simulink model "s_c_nbe_m1k_fulldb"  (R2016b) (continued)
== system s_c_nbe_m1k_fulldb == (continued)
  pipeline30/1(q) -> [force_gbe_txen]
  control/18(in_snap_stop_mux_sel) -> pipeline127/1(d)
  control/19(in_snap_reord_mux_we_sel) -> pipeline128/1(d)
  pipeline127/1(q) -> [snap_stop_mux_sel]
  pipeline128/1(q) -> [snap_reord_mux_we_sel]
  control/20(in_local_time_source) -> pipeline6/1(d)
  edge_detect6/1(out) -> pipeline164/1(d)
  pipeline164/1(q) -> [local_time_capture]
  pipeline6/1(q) -> [local_time_src]
  control/21(in_local_time_capture) -> edge_detect6/1(in)
  control/22(in_time_diff_check_en) -> pipeline62/1(d)
  pipeline62/1(q) -> [time_diff_check_en]
  control/23(in_auto_rst_enable) -> pipeline132/1(d)
  pipeline132/1(q) -> [auto_rst_enable]
  pipeline18/1(q) -> [pipeline_stall]
  control/24(in_pipeline_stall) -> pipeline18/1(d)
  Counter12/1 -> Slice13/1
  Slice13/1 -> edge_detect8/1(in)
  Logical15/1 -> Logical11/2, Counter12/1
  Logical11/1 -> [fault_detected]
  Register6/1 -> Inverter6/1
  Register/1 -> [synced], posedge_delay/1(In1)
  posedge_delay/1(Out1) -> [synced_delay]
  [board_in_fault] -> Inverter1/1
  Inverter1/1 -> Logical6/1
  [synced] -> Inverter2/1
  Inverter2/1 -> Counter1/2
  [pack_sync] -> Counter1/1
  Counter1/1 -> Slice16/1
  Slice16/1 -> edge_detect5/1(in)
  edge_detect8/1(out) -> Logical11/1
  [sys_relock] -> Delay57/1
  Inverter6/1 -> [board_in_fault]
  Delay57/1 -> Register6/2
  [synced_delay] -> edge_detect9/1(in)
  edge_detect9/1(out) -> Register6/3, Register6/1
  edge_detect5/1(out) -> [sync_timeout]
  [auto_rst_enable] -> Logical7/1
  [synced_delay] -> Logical7/2
  [fault_detected] -> Logical7/3
  [sync_timeout] -> Logical14/2
  [auto_rst_enable] -> Logical14/1
  Logical14/1 -> Logical8/2
  Logical7/1 -> Logical8/3
  pipeline23/1(q) -> [outfreq_stopmux]
  ct_debug/6(in_outfreq_stopmux) -> pipeline23/1(d)
  pipeline24/1(q) -> [out_stopmux]
  ct_debug/5(in_out_stopmux) -> pipeline24/1(d)
  pipeline55/1(q) -> [wrrd_stopmux]
  ct_debug/3(in_wrrd_stopmux) -> pipeline55/1(d)
  pipeline56/1(q) -> [in_stopmux]
  ct_debug/1(in_in_stopmux) -> pipeline56/1(d)
  pipeline122/1(q) -> [ct_snap_arm]
  pipeline22/1(q) -> edge_detect7/1
  ct_debug/8(in_snap_trig) -> pipeline22/1(d)
  ct_debug/7(in_snap_arm) -> pipeline122/1(d)
  edge_detect7/1 -> [ct_snap_trig]
  ct_debug/2(in_in_trigmux) -> pipeline150/1(d)
  pipeline150/1(q) -> [in_trigmux]
  ct_debug/4(in_obuf_stopmux) -> pipeline167/1(d)
  pipeline167/1(q) -> [obuf_stopmux]
  ct_debug/9(in_snap_wesel) -> pipeline81/1(d)
  pipeline81/1(q) -> [ct_snap_wesel]
  pipeline157/1(q) -> [ct_snap_trigsel]
  ct_debug/10(in_snap_trigsel) -> pipeline157/1(d)
  pipeline166/1(q) -> [ct_snap_circsel]
  ct_debug/11(in_snap_circsel) -> pipeline166/1(d)
  bus_create2/1(bus_out) -> [tvgtone_level0]
  bus_create3/1(bus_out) -> [tvgtone_level1]
  tvg_tone_levels/2(in_p0i) -> bus_create2/2(in2)
  tvg_tone_levels/3(in_p1r) -> bus_create3/1(in1)
  tvg_tone_levels/4(in_p1i) -> bus_create3/2(in2)
  tvg_tone_levels/1(in_p0r) -> bus_create2/1(in1)
  tvg_tone_targets/1(in_p0) -> Slice18/1
  tvg_tone_targets/2(in_p1) -> Slice19/1
  Constant51/1 -> tvg_tone_targets/1(sim_1)
  Constant52/1 -> tvg_tone_targets/2(sim_2)
  Constant37/1 -> tvg_tone_levels/4(sim_4)
  Constant8/1 -> tvg_tone_levels/1(sim_1)
  Constant27/1 -> tvg_tone_levels/3(sim_3)
  Constant9/1 -> tvg_tone_levels/2(sim_2)
  we_delay49/1(q) -> [ct_tag_insert_sel]
  edge_detect2/1 -> [ct_rst_err_latch]
  we_delay47/1(q) -> edge_detect2/1
  we_delay48/1(q) -> [ct_fake_data_error]
  we_delay13/1(q) -> [ct_tvg_en]
  we_delay50/1(q) -> [ct_tvg_en2]
  Constant12/1 -> ct_control0/5(sim_5)
  Constant21/1 -> ct_control0/4(sim_4)
  Constant20/1 -> ct_control0/3(sim_3)
  Constant22/1 -> ct_control0/2(sim_2)
  Constant15/1 -> ct_control0/1(sim_1)
  ct_control0/1(in_tvg_en) -> we_delay13/1(d)
  ct_control0/2(in_tvg_en2) -> we_delay50/1(d)
  ct_control0/3(in_rst_err_latch) -> we_delay47/1(d)
  ct_control0/4(in_fake_error) -> we_delay48/1(d)
  ct_control0/5(in_tag_insert) -> we_delay49/1(d)
  we_delay126/1(q) -> [obuf_read_gap]
  ct_control0/6(in_obuf_read_gap) -> we_delay126/1(d)
  Constant30/1 -> ct_control0/6(sim_6)
  we_delay41/1(q) -> [ct_num_xeng]
  we_delay112/1(q) -> [ct_num_xeng_recip]  "NUM_X_RECIP"
  ct_control1/2(in_num_x_recip) -> we_delay112/1(d)
  ct_control1/1(in_num_x) -> we_delay41/1(d)
  we_delay114/1(q) -> [ct_x_per_board]
  ct_control1/3(in_x_per_board) -> we_delay114/1(d)
  we_delay113/1(q) -> [ct_chans_per_board]
  ct_control2/1(in_chans_per_x) -> we_delay39/1(d)
  ct_control2/2(in_chans_per_board) -> we_delay113/1(d)
  we_delay115/1(q) -> [ct_x_per_board_recip]
  ct_control1/4(in_x_per_board_recip) -> we_delay115/1(d)
  we_delay38/1(q) -> [ct_num_x_boards]
  ct_control3/1(in_num_x_boards) -> we_delay38/1(d)
  we_delay37/1(q) -> [ct_num_x_boards_recip]
  ct_control3/2(in_num_x_boards_recip) -> we_delay37/1(d)
  ct_control3/3(in_chans_per_x_recip) -> we_delay124/1(d)
  Constant49/1 -> ct_control1/1(sim_1)
  Constant53/1 -> ct_control1/2(sim_2)
  Constant33/1 -> ct_control1/3(sim_3)
  Constant23/1 -> ct_control2/1(sim_1)
  Constant50/1 -> ct_control2/2(sim_2)
  Constant54/1 -> ct_control1/4(sim_4)
  Constant32/1 -> ct_control3/1(sim_1)
  Constant14/1 -> ct_control3/2(sim_2)
  Constant44/1 -> ct_control3/3(sim_3)
  Slice18/1 -> [tvgtone_p0]
  Slice19/1 -> [tvgtone_p1]
  Slice36/1 -> we_delay116/1(d)
  Constant38/1 -> ct_control4/1(sim_1)
  ct_control4/1(in_ct_board_offset) -> Slice36/1
  ct_control5/1(in_ct_freq_gen_offset) -> we_delay117/1(d)
  Constant39/1 -> ct_control5/1(sim_1)
  we_delay116/1(q) -> [ct_board_hmc_offset]
  we_delay117/1(q) -> [ct_freq_gen_offset]
  we_delay124/1(q) -> [ct_chans_per_x_recip]
  we_delay39/1(q) -> [ct_chans_per_x]  "PER_X"
  pipeline196/1(q) -> ddc_dvalid_cnt/1(out_reg)
  pipeline197/1(q) -> ddc_sync_cnt/1(out_reg)
  pipeline198/1(q) -> DDC/8(scale1)
  pipeline199/1(q) -> DDC/5(scale0)
  trig_time_msw/1(in_msw) -> pipeline213/1(d)
  trig_time_lsw/1(in_lsw) -> pipeline214/1(d)
  Concat13/1 -> Relational10/1
  pipeline213/1(q) -> Concat13/1
  pipeline214/1(q) -> Concat13/2
  [time80_48] -> Relational10/2
  pipeline215/1(q) -> bus_expand18/1(bus_in)
  [valid80] -> Slice5/1
  Slice14/1 -> pipeline211/1(d)
  [time80_48] -> Slice14/1
  pipeline211/1(q) -> snap_adc0/13(extra_timestamp)
  [d80_adc0] -> pipeline215/1(d)
  bus_expand18/4(out5) -> snap_adc0/4(in_p0_d3)
  bus_expand18/5(out4) -> snap_adc0/5(in_p0_d4)
  bus_expand18/1(msb_out8) -> Gateway Out3/1, snap_adc0/1(in_p0_d0)
  bus_expand18/2(out7) -> Gateway Out23/1, snap_adc0/2(in_p0_d1)
  bus_expand18/3(out6) -> snap_adc0/3(in_p0_d2)
  bus_expand18/6(out3) -> snap_adc0/6(in_p0_d5)
  bus_expand18/7(out2) -> snap_adc0/7(in_p0_d6)
  bus_expand18/8(lsb_out1) -> snap_adc0/8(in_p0_d7)
  pipeline202/1(q) -> snap_adc0/11(we)
  Relational10/1 -> Logical12/1
  [valid80] -> pipeline162/1(d)
  Logical12/1 -> snap_adc0/12(trig)
  pipeline162/1(q) -> Logical12/2
  [pfbin_sync] -> Delay21/1
  [ddc_sync_in] -> pipeline97/1(d)
  pipeline68/1(q) -> pipeline66/1(d)
  pipeline83/1(q) -> pipeline59/1(d)
  Slice5/1 -> pipeline68/1(d), pipeline202/1(d)
  [sync80] -> pipeline83/1(d)
  [ddc_pol1_in] -> pipeline93/1(d)
  Slice17/1 -> pipeline121/1(d)
  Slice15/1 -> Convert1/1
  [local_time_capture] -> Delay31/1
  pipeline19/1(q) -> Slice17/1, Slice15/1
  [local_time_src] -> Mux/1
  [time80_48] -> Delay14/1
  [valid80] -> Delay14/2
  Delay14/1 -> Register1/1, AddSub/2
  [pfb_time] -> Delay10/1
  [pfb_dv] -> Delay10/2
  Delay11/1 -> Register3/1, AddSub/1
  [flag_dvalid] -> Delay11/2
  [pack_sync_time] -> Delay11/1
  Convert1/1 -> pipeline123/1(d)
  AddSub/1 -> pipeline140/1(d), Relational4/1
  Relational4/1 -> Logical/1
  Logical/1 -> [time_diff_error]
  time_check/1(in_max_difference) -> pipeline139/1(d)
  [time_diff_check_en] -> Logical/2
  Delay31/1 -> Register3/2, Register2/2, Register4/2, Register1/2
  Delay10/1 -> Register2/1
  [spead_timestamp] -> Register4/1
  Register4/1 -> Mux/2
  Register1/1 -> Mux/3
  Register2/1 -> Mux/4
  Register3/1 -> Mux/5
  Mux/1 -> pipeline19/1(d)
  [cnt_rst] -> Subsystem2/1(rst)
  [synced] -> Delay38/1
  [sync_armed] -> Delay39/1
  [board_in_fault] -> Delay48/1
  [q_sync] -> Subsystem19/5(in3)
  [pfb_sync] -> Subsystem19/3(in1)
  [fdfs_sync] -> Subsystem19/4(in2)
  [sync80] -> Subsystem19/2(in0)
  [cnt_rst] -> Subsystem19/1(rst)
  Subsystem19/2(out1) -> pipeline35/1(d)
  Subsystem19/1(out0) -> pipeline34/1(d)
  Subsystem19/3(out2) -> pipeline36/1(d)
  [pack_sync] -> Subsystem12/4(in2)
  [flag_sync] -> Subsystem12/3(in1)
  [ct_sync] -> Subsystem12/2(in0)
  [cnt_rst] -> Subsystem12/1(rst)
  [synced_delay] -> Subsystem2/2(armed)
  [sync_timeout] -> Subsystem2/3(in1)
  [time_diff_error] -> Subsystem2/4(in2)
  [up_timestep_error] -> Subsystem2/5(in3)
  [cd_error] -> Subsystem2/6(in4)
  [ct_error] -> Subsystem2/7(in5)
  Delay48/1 -> pipeline5/1(d)
  Delay38/1 -> pipeline10/1(d)
  Delay39/1 -> pipeline11/1(d)
  Subsystem12/1(out0) -> pipeline16/1(d)
  Subsystem12/2(out1) -> pipeline20/1(d)
  Subsystem12/3(out2) -> pipeline21/1(d)
  Subsystem2/1(Out1) -> pipeline25/1(d)
  Subsystem2/2(Out2) -> pipeline26/1(d)
  Subsystem2/3(Out3) -> pipeline27/1(d)
  [synced_delay] -> Delay58/1
  Delay58/1 -> pipeline4/1(d)
  Subsystem19/4(out3) -> pipeline37/1(d)
  Subsystem2/4(Out4) -> pipeline32/1(d)
  Subsystem2/5(Out5) -> pipeline33/1(d)
  [clr_status] -> Subsystem3/1(rst)
  Slice6/1 -> Subsystem3/2(in0)
  [pfb_of] -> Slice6/1, Slice20/1
  Slice20/1 -> Subsystem3/3(in1)
  [pfb_sync] -> Subsystem3/4(in2)
  Subsystem3/3(out2) -> pipeline193/1(d)
  Subsystem3/1(out0) -> pipeline195/1(d)
  Subsystem3/2(out1) -> pipeline194/1(d)
  Constant17/1 -> [pfb_of]
  Counter7/1 -> pipeline191/1(d)
  [pfb_sync] -> pipeline126/1(d)
  [cnt_rst] -> pipeline129/1(d)
  pipeline191/1(q) -> nb_sync_cnt/1(out_reg)
  pipeline193/1(q) -> pfb_status/3(out_sync_cnt)
  pipeline194/1(q) -> pfb_status/2(out_pol1_or_err_cnt)
  pipeline195/1(q) -> pfb_status/1(out_pol0_or_err_cnt)
  Delay72/1 -> [flag_sync]
  Delay75/1 -> [flag_misc]
  Delay73/1 -> [flag_dvalid]
  Delay74/1 -> [flag_time]
  Delay43/1 -> time_gen1/4(rd_sync_in)
  Delay59/1 -> time_gen1/6(rd_misc_in)
  Delay/1 -> Delay43/1  "gapsync"
  Delay3/1 -> Delay47/1  "gapdata"
  Mux1/1 -> Delay59/1  "gapvalid"
  [time80] -> Slice2/1
  Delay47/1 -> time_gen1/5(rd_en)
  time_gen1/1(rd_sync_out) -> Delay72/1
  time_gen1/4(rd_misc_out) -> Delay75/1
  time_gen1/2(rd_dvalid) -> Delay73/1
  time_gen1/3(rd_val_out) -> Delay74/1
  Slice2/1 -> time_gen1/3(wr_val_in)
  [ct_tvg_en2] -> Mux1/1
  Delay17/1 -> Mux1/2
  Delay18/1 -> tvg/1(sync), Delay/1
  Delay16/1 -> tvg/2(dv), Delay3/1
  tvg/1(data256) -> Mux1/3
  [flag_dvalid] -> Subsystem13/1(In1)
  Subsystem13/1(Out1) -> pipeline186/1(d)
  [s256_v] -> Subsystem11/1(In1)
  Subsystem11/1(Out1) -> pipeline187/1(d)
  pipeline38/1(q) -> Convert3/1
  pipeline46/1(q) -> [ip_per_x]
  pipeline47/1(q) -> [num_x]
  x_setup/1(in_f_per_x) -> pipeline38/1(d)
  x_setup/2(in_ip_per_x) -> pipeline46/1(d)
  x_setup/3(in_num_x) -> pipeline47/1(d)
  pipeline52/1(q) -> [pack_snap_arm]
  x_setup/4(in_snap_arm) -> pipeline52/1(d)
  pipeline48/1(q) -> [pktstart_sel]
  x_setup/5(in_pktstart_mux) -> pipeline48/1(d)
  [s256_v] -> pack_block_check/1(dv)
  [cnt_rst] -> Subsystem14/1(rst)
  Subsystem14/1(out0) -> pipeline188/1(d)
  pack_block_check/1(error) -> [pack_dv_err], Subsystem14/2(in0)
  pipeline49/1(q) -> [pack_mux]
  x_setup/6(in_pack_mux) -> pipeline49/1(d)
  Convert3/1 -> [f_per_x]
  [ct_d256] -> Delay17/1
  [ct_dv] -> Delay16/1
  [ct_sync] -> Delay18/1
  x_setup/7(in_pktoffset_sel) -> pipeline54/1(d)
  pipeline54/1(q) -> [pktoffset_sel]
  iptx_base/1(in_reg) -> pipeline53/1(d)
  pipeline53/1(q) -> [tx_ip_base]
  tx_metadata/1(in_porttx) -> pipeline50/1(d)
  tx_metadata/2(in_board_id) -> pipeline51/1(d)
  pipeline50/1(q) -> [tx_port]
  pipeline51/1(q) -> [tx_board_id]
  Delay64/1 -> Delay68/1
  Delay4/1 -> Delay5/1
  Delay5/1 -> Delay60/1
  Delay60/1 -> Delay64/1
  Delay68/1 -> [flag_pktstart]
  Delay1/1 -> Delay6/1
  Delay6/1 -> Delay61/1
  Delay65/1 -> Delay69/1
  Delay69/1 -> [flag_pktfreq]
  Delay61/1 -> Delay65/1
  Delay15/1 -> Delay7/1
  Delay7/1 -> Delay62/1
  Delay62/1 -> Delay66/1
  Delay66/1 -> Delay70/1
  Delay70/1 -> [flag_pktxindex]
  Delay19/1 -> Delay20/1
  Delay20/1 -> Delay63/1
  Delay63/1 -> Delay67/1
  Delay67/1 -> Delay71/1
  Delay71/1 -> [flag_pktxoffset]
  [ct_pkt_x_index] -> Delay15/1
  [ct_pkt_x_offset] -> Delay19/1
  [ct_pktfreq] -> Delay1/1
  [ct_pktstart] -> Delay4/1
  [flag_sync] -> packetiser/1(sync_in)
  [flag_dvalid] -> packetiser/2(en)
  [flag_pktstart] -> packetiser/5(pkt_start)
  [flag_pktxoffset] -> packetiser/7(pkt_x_offset)
  [tx_ip_base] -> packetiser/9(base_ip)
  [tx_port] -> packetiser/10(base_port)
  Constant3/1 -> concat1/2
  [flag_time] -> concat1/1
  [flag_misc] -> packetiser/3(din)
  concat1/1 -> [pack_sync_time], packetiser/4(timestamp)
  Delay82/1 -> [s256_d]
  Delay83/1 -> [s256_v]
  Delay84/1 -> [s256_eof]
  Delay85/1 -> [s256_of]
  Delay86/1 -> [s256_ip]
  Delay87/1 -> [s256_port]
  Delay88/1 -> [pack_sync]
  packetiser/1(dout) -> Delay82/1
  packetiser/2(dvalid) -> Delay83/1
  packetiser/3(eof) -> Delay84/1
  packetiser/4(overflow) -> Delay85/1
  packetiser/5(destination_ip) -> Delay86/1
  packetiser/6(destination_port) -> Delay87/1
  packetiser/7(sync) -> Delay88/1
  [flag_pktfreq] -> Delay77/1
  Delay89/1 -> [s256_pktfreq]
  Delay77/1 -> Delay89/1
  [flag_pktxindex] -> Delay78/1
  Delay78/1 -> Delay90/1
  Delay90/1 -> [s256_pktxindex]
  [flag_pktxoffset] -> Delay79/1
  Delay79/1 -> Delay91/1
  Delay91/1 -> [s256_pktxoffset]
  [tx_board_id] -> packetiser/8(board_id)
  [flag_pktstart] -> Delay80/1
  Delay80/1 -> Delay92/1
  Delay92/1 -> [s256_pktstart]
  [sync80] -> time_gen1/1(wr_sync_in)
  [valid80] -> time_gen1/2(wr_en)
  [time80_48] -> Subsystem/1(time)
  [sync80] -> Subsystem/2(sync)
  [valid80] -> Subsystem/3(dv)
  Subsystem/1(msw) -> pipeline184/1(d)
  Subsystem/2(ls32) -> pipeline185/1(d)
  [flag_pktxindex] -> packetiser/6(pkt_x_index)
  [flag_time] -> Delay81/1
  Delay81/1 -> Delay93/1
  Delay93/1 -> [s256_time]
  time_gen1/5(epoch) -> Delay76/1
  Slice4/1 -> pipeline182/1(d)
  Slice11/1 -> pipeline183/1(d)
  Delay76/1 -> Concat10/1
  Constant16/1 -> Concat10/2
  Concat10/1 -> Slice4/1, Slice11/1, [flag_epoch]
  [flag_pktfreq] -> Subsystem27/3(value)
  [cnt_rst] -> Subsystem27/1(rst)
  [flag_dvalid] -> Subsystem27/2(en)
  Subsystem27/1(current) -> pipeline170/1(d)
  Subsystem27/3(min) -> pipeline172/1(d)
  Subsystem27/2(max) -> pipeline171/1(d)
  [flag_pktxindex] -> Subsystem28/3(value)
  [cnt_rst] -> Subsystem28/1(rst)
  [flag_dvalid] -> Subsystem28/2(en)
  Subsystem28/1(current) -> pipeline173/1(d)
  Subsystem28/3(min) -> pipeline175/1(d)
  Subsystem28/2(max) -> pipeline174/1(d)
  [flag_pktxoffset] -> Subsystem29/3(value)
  [cnt_rst] -> Subsystem29/1(rst)
  [flag_dvalid] -> Subsystem29/2(en)
  Subsystem29/1(current) -> pipeline176/1(d)
  Subsystem29/3(min) -> pipeline178/1(d)
  Subsystem29/2(max) -> pipeline177/1(d)
  [flag_time] -> Subsystem30/3(value)
  [cnt_rst] -> Subsystem30/1(rst)
  [flag_pktstart] -> Subsystem30/2(en)
  Subsystem30/1(current) -> pipeline179/1(d)
  Subsystem30/3(min) -> pipeline181/1(d)
  Subsystem30/2(max) -> pipeline180/1(d)
  pipeline170/1(q) -> flag_pktfreq_stats/1(out_current)
  pipeline171/1(q) -> flag_pktfreq_stats/2(out_max)
  pipeline172/1(q) -> flag_pktfreq_stats/3(out_min)
  pipeline175/1(q) -> flag_pktxindex_stats/3(out_min)
  pipeline174/1(q) -> flag_pktxindex_stats/2(out_max)
  pipeline173/1(q) -> flag_pktxindex_stats/1(out_current)
  pipeline176/1(q) -> flag_pktxoffset_stats/1(out_current)
  pipeline177/1(q) -> flag_pktxoffset_stats/2(out_max)
  pipeline178/1(q) -> flag_pktxoffset_stats/3(out_min)
  pipeline181/1(q) -> flag_time_stats/3(out_min)
  pipeline180/1(q) -> flag_time_stats/2(out_max)
  pipeline179/1(q) -> flag_time_stats/1(out_current)
  pipeline182/1(q) -> flag_epoch_lsw/1(out_reg)
  pipeline183/1(q) -> flag_epoch_msw/1(out_reg)
  pipeline184/1(q) -> sync_time_msw/1(out_reg)
  pipeline185/1(q) -> sync_time_lsw/1(out_reg)
  pipeline186/1(q) -> pack_in_dv_rate/1(out_dv_rate)
  pipeline187/1(q) -> pack_out_dv_rate/1(out_dv_rate)
  pipeline188/1(q) -> pack_dv_err/1(out_dvblock_err_cnt)
  [pfbin_sync] -> Logical9/2
  [sys_rst] -> Logical9/1
  Logical9/1 -> pipeline147/1(d)
  [osc_cos_ddc0] -> pipeline92/1(d)
  [osc_cos_ddc0] -> pipeline94/1(d)
  [pfbin_d0] -> Delay9/1
  [pfbin_dv] -> Delay2/1
  Delay21/1 -> pipeline144/1(d)
  [ddc_pol0] -> nb_pfb/2(pol0)
  [ddc_pol1] -> nb_pfb/3(pol1)
  nb_pfb/2(pol0_out) -> [pfb_d144_0]
  nb_pfb/3(pol1_out) -> [pfb_d144_1]
  [ddc_sync] -> nb_pfb/1(sync)
  nb_pfb/1(sync_out) -> [pfb_sync]
  [ddc_dvalid] -> nb_pfb/4(dvalid)
  nb_pfb/4(dv_out) -> [pfb_dv]
  [ddc_dv_in] -> pipeline96/1(d)
  [ddc_pol0_in] -> pipeline95/1(d)
  [pfbin_d1] -> Delay22/1
  [pfbin_dv] -> cwg1/6(en)
  Delay22/1 -> pipeline149/1(d)
  Gateway Out3/1 -> To Workspace4/1
  Gateway Out23/1 -> To Workspace23/1
  tl_fd0/2(enable) -> phase_compensation0/5(load_value)
  tl_fd1/1(value) -> phase_compensation1/4(delay_value)
  tl_fd1/2(enable) -> phase_compensation1/5(load_value)
  tl_fd0/1(value) -> phase_compensation0/4(delay_value)
  [clr_status] -> tl_fd1/1(rst), tl_fd0/1(rst)
  phase_compensation0/1(sync_out) -> [fdfs_sync]
  phase_compensation1/1(sync_out) -> Terminator24/1
  phase_compensation0/2(dout) -> [fdfs_d144_0]
  phase_compensation1/2(dout) -> [fdfs_d144_1]
  phase_compensation0/3(dvalid) -> [fdfs_dv]
  phase_compensation1/3(dvalid) -> Terminator25/1
  [ld_val_fd0] -> tl_fd0/3(load_value)
  [ld_val_fd1] -> tl_fd1/3(load_value)
  bus_expand16/1(msb_out2) -> tl_fd0/5(din)
  tl_fd0/5(dvalid) -> phase_compensation0/3(en)
  tl_fd0/3(sync_out) -> phase_compensation0/1(sync_in)
  tl_fd0/4(dout) -> phase_compensation0/2(din)
  tl_fd1/4(dout) -> phase_compensation1/2(din)
  bus_expand16/2(lsb_out1) -> tl_fd1/5(din)
  Constant66/1 -> Concat2/2
  Concat2/1 -> tl_fd1/2(current_time), tl_fd0/2(current_time), [pfb_time]
  Counter2/1 -> pipeline41/1(d)
  Constant65/1 -> eq1/2(data_in)
  Constant24/1 -> eq1/3(we)
  Delay53/1 -> quant1/1(sync_in)
  Delay37/1 -> quant1/2(din)
  eq1/1(data_out) -> we_delay28/1(d)
  Delay50/1 -> quant1/3(en)
  Counter4/1 -> pipeline29/1(d)
  Constant63/1 -> eq0/2(data_in)
  Constant64/1 -> eq0/3(we)
  Delay51/1 -> quant0/1(sync_in)
  Delay35/1 -> quant0/2(din)
  Delay52/1 -> quant0/3(en)
  quant0/1(sync_out) -> [q_sync]
  quant0/2(dout) -> [q_d64_0]
  quant0/3(dvalid) -> [q_dv]
  quant0/4(of) -> [q_of0]
  quant1/2(dout) -> [q_d64_1]
  quant1/1(sync_out) -> Terminator19/1
  quant1/3(dvalid) -> Terminator21/1
  quant1/4(of) -> [q_of1]
  pipeline29/1(q) -> eq0/1(addr)
  pipeline41/1(q) -> eq1/1(addr)
  [fdfs_sync] -> Delay51/1, pipeline45/1(d)
  [fdfs_d144_0] -> Delay35/1
  [fdfs_dv] -> Delay52/1, pipeline42/1(d)
  pipeline42/1(q) -> Counter4/2
  pipeline45/1(q) -> Counter4/1
  pipeline189/1(q) -> Counter2/2
  [fdfs_sync] -> Delay53/1, pipeline200/1(d)
  [fdfs_d144_1] -> Delay37/1
  [fdfs_dv] -> Delay50/1, pipeline189/1(d)
  pipeline200/1(q) -> Counter2/1
  [clr_status] -> Subsystem15/1(rst)
  tl_fd0/7(misco) -> Terminator3/1
  tl_fd0/6(time) -> Terminator4/1
  tl_fd1/6(time) -> Terminator17/1
  tl_fd1/3(sync_out) -> phase_compensation1/1(sync_in)
  tl_fd1/5(dvalid) -> phase_compensation1/3(en)
  tl_fd1/7(misco) -> Terminator20/1
  Constant26/1 -> tl_fd0/7(misci)
  Constant29/1 -> tl_fd1/7(misci)
  time_gen/1(rd_sync_out) -> tl_fd1/4(sync_in), tl_fd0/4(sync_in)
  time_gen/2(rd_dvalid) -> tl_fd1/6(en), tl_fd0/6(en)
  time_gen/3(rd_val_out) -> Concat2/1
  time_gen/4(rd_misc_out) -> bus_expand16/1(bus_in)
  [sync80] -> pipeline207/1(d)
  [valid80] -> pipeline208/1(d)
  [pfb_sync] -> pipeline210/1(d)
  [pfb_dv] -> pipeline212/1(d)
  [pfb_d144_1] -> pipeline231/1(d)
  [pfb_d144_0] -> pipeline228/1(d)
  Concat1/1 -> time_gen/6(rd_misc_in)
  [time80] -> pipeline209/1(d)
  Subsystem15/1(out0) -> we_delay18/1(d)
  Relational/1 -> Subsystem15/2(in0)
  Constant4/1 -> Relational/2
  [q_of0] -> Relational/1
  Relational3/1 -> Subsystem15/3(in1)
  [q_of1] -> Relational3/1
  Constant25/1 -> Relational3/2
  Subsystem15/2(out1) -> we_delay19/1(d)
  [tl_cd0_arm] -> tl_fd0/8(arm)
  [tl_cd0_load_immediate] -> tl_fd0/9(load_immediate)
  [tl_cd0_load_time] -> tl_fd0/10(load_time)
  [tl_cd1_arm] -> tl_fd1/8(arm)
  [tl_cd1_load_immediate] -> tl_fd1/9(load_immediate)
  [tl_cd1_load_time] -> tl_fd1/10(load_time)
  pipeline207/1(q) -> time_gen/1(wr_sync_in)
  pipeline208/1(q) -> time_gen/2(wr_en)
  pipeline209/1(q) -> time_gen/3(wr_val_in)
  pipeline210/1(q) -> time_gen/4(rd_sync_in)
  pipeline212/1(q) -> time_gen/5(rd_en)
  pipeline228/1(q) -> Concat1/1
  pipeline231/1(q) -> Concat1/2
  Concat4/1 -> pipeline133/1(d)
  Concat9/1 -> pipeline130/1(d)
  fd0_phase/1(in_initial) -> Reinterpret10/1
  fd0_delta_delay/1(in_delta) -> Reinterpret9/1
  fd0_phase/2(in_delta) -> Reinterpret11/1
  Reinterpret16/1 -> Concat4/1
  Reinterpret9/1 -> Concat4/2
  Reinterpret10/1 -> Concat4/3
  Reinterpret11/1 -> Concat4/4
  fd1_phase/1(in_initial) -> Reinterpret13/1
  fd1_delta_delay/1(in_delta) -> Reinterpret12/1
  fd1_phase/2(in_delta) -> Reinterpret14/1
  Reinterpret12/1 -> Concat9/2
  Reinterpret13/1 -> Concat9/3
  Reinterpret14/1 -> Concat9/4
  Reinterpret15/1 -> Concat9/1
  fd0_delay/1(in_initial) -> Reinterpret16/1
  fd1_delay/1(in_initial) -> Reinterpret15/1
  [ct_dv] -> Subsystem21/1(In1)
  Subsystem21/1(Out1) -> we_delay22/1(d)
  [ct_pktstart] -> we_delay3/1(d)
  [ct_dv] -> we_delay4/1(d)
  we_delay3/1(q) -> pktlen_check1/1(pkt_start)
  we_delay4/1(q) -> dv_block_check1/1(dv), pktlen_check1/2(dv)
  pktlen_check1/1(error) -> we_delay6/1(d)
  dv_block_check1/1(error) -> we_delay5/1(d)
  [cnt_rst] -> Subsystem20/1(rst)
  we_delay6/1(q) -> [ct_pktlen_err], Subsystem20/2(in0)
  we_delay5/1(q) -> [ct_dvblk_err], Subsystem20/3(in1)
  Subsystem20/1(out0) -> we_delay20/1(d)
  Subsystem20/2(out1) -> we_delay21/1(d)
  [q_dv] -> Subsystem18/1(In1)
  Subsystem18/1(Out1) -> we_delay23/1(d)
  [q_sync] -> we_delay14/1(d)
  [q_dv] -> we_delay15/1(d)
  [q_d64_0] -> we_delay16/1(d)
  [q_d64_1] -> we_delay17/1(d)
  hmc_ct/1(out_sync) -> we_delay12/1(d)
  hmc_ct/2(out_dv) -> we_delay11/1(d)
  hmc_ct/3(out_d256) -> we_delay10/1(d)
  hmc_ct/4(pkt_start) -> we_delay9/1(d)
  hmc_ct/5(freq_id) -> we_delay8/1(d)
  hmc_ct/6(x_index) -> we_delay7/1(d)
  hmc_ct/7(x_pkt_index) -> we_delay2/1(d)
  hmc_ct/8(error0) -> we_delay1/1(d)
  [cnt_rst] -> Subsystem16/1(rst)
  [q_sync] -> Subsystem16/2(in0)
  Constant67/1 -> Subsystem16/3(in1)
  Constant68/1 -> Subsystem16/4(in2)
  [ct_sync] -> Subsystem16/5(in3)
  Subsystem16/1(out0) -> we_delay24/1(d)
  Subsystem16/2(out1) -> we_delay25/1(d)
  Subsystem16/3(out2) -> we_delay26/1(d)
  Subsystem16/4(out3) -> we_delay27/1(d)
  Convert2/1 -> [cd_bypass]
  pipeline59/1(q) -> snap_adc0/9(in_sync)
  pipeline66/1(q) -> snap_adc0/10(in_dv)
  pipeline8/1(q) -> [unpack_dv]
  pipeline17/1(q) -> [unpack_timestamp]
  pipeline14/1(q) -> [tvg_adc0_en]
  pipeline72/1(q) -> bus_expand4/1(bus_in)
  From8/1 -> Slice3/1
  Slice1/1 -> pipeline71/1(d)
  From33/1 -> Slice1/1
  pipeline71/1(q) -> snap_adc1/13(extra_timestamp)
  From35/1 -> pipeline72/1(d)
  bus_expand4/4(out5) -> snap_adc1/4(in_p0_d3)
  bus_expand4/5(out4) -> snap_adc1/5(in_p0_d4)
  bus_expand4/1(msb_out8) -> snap_adc1/1(in_p0_d0)
  bus_expand4/2(out7) -> snap_adc1/2(in_p0_d1)
  bus_expand4/3(out6) -> snap_adc1/3(in_p0_d2)
  bus_expand4/6(out3) -> snap_adc1/6(in_p0_d5)
  bus_expand4/7(out2) -> snap_adc1/7(in_p0_d6)
  bus_expand4/8(lsb_out1) -> snap_adc1/8(in_p0_d7)
  pipeline67/1(q) -> snap_adc1/11(we)
  pipeline77/1(q) -> pipeline76/1(d)
  pipeline79/1(q) -> snap_adc1/12(trig), pipeline75/1(d)
  Slice3/1 -> pipeline67/1(d), pipeline77/1(d)
  From34/1 -> pipeline79/1(d)
  pipeline75/1(q) -> snap_adc1/9(in_sync)
  pipeline76/1(q) -> snap_adc1/10(in_dv)
  pipeline13/1(q) -> [osc_cos_ddc0]
  Gateway Out5/1 -> To Workspace5/1
  [q_d64_0] -> bus_expand31/1(bus_in)
  [q_dv] -> Gateway Out5/1
  Gateway Out6/1 -> To Workspace6/1
  [q_sync] -> Gateway Out6/1
  Gateway Out7/1 -> To Workspace7/1
  bus_expand31/1(msb_out8) -> Gateway Out7/1
  Gateway Out8/1 -> To Workspace8/1
  Gateway Out9/1 -> To Workspace9/1
  bus_expand31/3(out6) -> Gateway Out9/1
  Gateway Out10/1 -> To Workspace10/1
  bus_expand31/5(out4) -> Gateway Out10/1
  Gateway Out11/1 -> To Workspace11/1
  bus_expand31/7(out2) -> Gateway Out11/1
  Gateway Out12/1 -> To Workspace12/1
  bus_expand31/4(out5) -> Gateway Out12/1
  Gateway Out13/1 -> To Workspace13/1
  bus_expand31/6(out3) -> Gateway Out13/1
  Gateway Out14/1 -> To Workspace14/1
  bus_expand31/8(lsb_out1) -> Gateway Out14/1
  bus_expand31/2(out7) -> Gateway Out8/1
  Gateway Out20/1 -> To Workspace20/1
  bus_expand32/1(msb_out8) -> Gateway Out20/1
  Gateway Out21/1 -> To Workspace15/1
  Gateway Out22/1 -> To Workspace16/1
  bus_expand32/3(out6) -> Gateway Out22/1
  Gateway Out15/1 -> To Workspace17/1
  bus_expand32/5(out4) -> Gateway Out15/1
  Gateway Out16/1 -> To Workspace18/1
  bus_expand32/7(out2) -> Gateway Out16/1
  Gateway Out17/1 -> To Workspace19/1
  bus_expand32/4(out5) -> Gateway Out17/1
  Gateway Out18/1 -> To Workspace21/1
  bus_expand32/6(out3) -> Gateway Out18/1
  Gateway Out19/1 -> To Workspace22/1
  bus_expand32/8(lsb_out1) -> Gateway Out19/1
  bus_expand32/2(out7) -> Gateway Out21/1
  [fdfs_d144_0] -> bus_expand32/1(bus_in)
  Gateway Out24/1 -> To Workspace24/1
  [fdfs_dv] -> Gateway Out24/1
  Gateway Out25/1 -> To Workspace25/1
  [fdfs_sync] -> Gateway Out25/1
  eq0/1(data_out) -> we_delay29/1(d)
  impulse_gen0/1(sync_out) -> [sync80]
  impulse_gen0/3(dvalid) -> [valid80]
  impulse_gen0/2(dout) -> [d80_adc0]
  [ld_val0] -> pipeline296/1(d)
  [clr_status] -> pipeline278/1(d), pipeline298/1(d)
  tl_cd0/6(time) -> [time80_tl_cd0]
  Constant74/1 -> Concat15/2
  Concat15/1 -> pipeline277/1(d), pipeline297/1(d)
  [time80] -> Concat15/1
  pipeline298/1(q) -> tl_cd0/1(rst)
  pipeline297/1(q) -> tl_cd0/2(current_time)
  pipeline296/1(q) -> tl_cd0/3(load_value)
  pipeline295/1(q) -> tl_cd0/4(sync_in)
  pipeline294/1(q) -> tl_cd0/5(din)
  pipeline299/1(q) -> tl_cd0/6(en)
  [sync80] -> pipeline283/1(d), pipeline295/1(d)
  [valid80] -> pipeline281/1(d), pipeline299/1(d)
  Constant13/1 -> tl_cd0/7(misci)
  tl_cd0/7(misco) -> Terminator6/1
  tl_cd0/3(sync_out) -> [sync_tl_cd0]
  tl_cd0/5(dvalid) -> [dvalid_tl_cd0]
  tl_cd0/4(dout) -> [dout_tl_cd0]
  tl_cd0/2(enable) -> [en_tl_cd0]
  tl_cd0/1(value) -> [delay_tl_cd0]
  pipeline307/1(q) -> tl_cd0/10(load_time)
  pipeline306/1(q) -> tl_cd0/9(load_immediate)
  pipeline305/1(q) -> tl_cd0/8(arm)
  [tl_cd0_arm] -> pipeline305/1(d)
  [tl_cd0_load_immediate] -> pipeline306/1(d)
  [tl_cd0_load_time] -> pipeline307/1(d)
  [d80_adc0] -> pipeline294/1(d)
  [dvalid_tl_cd0] -> Delay117/1
  [sync_tl_cd0] -> Delay120/1
  [en_tl_cd0] -> Delay115/1
  [delay_tl_cd0] -> Delay116/1
  Concat5/1 -> time_gen_cd/6(rd_misc_in)
  time_gen_cd/4(rd_misc_out) -> bus_expand7/1(bus_in)
  [sync_tl_cd0] -> time_gen_cd/1(wr_sync_in)
  [dvalid_tl_cd0] -> time_gen_cd/2(wr_en)
  [hmc_sync_p0] -> time_gen_cd/4(rd_sync_in)
  [hmc_dvalid] -> time_gen_cd/5(rd_en)
  time_gen_cd/1(rd_sync_out) -> [tg_rd_sync]
  time_gen_cd/2(rd_dvalid) -> [tg_rd_dvalid]
  [dvalid_tl_bs0] -> wb_prog_delay/4(en)
  [delay_tl_bs0] -> wb_prog_delay/2(delay_val)
  [delay_tl_sync0] -> wb_prog_delay/1(sync)
  [en_tl_bs0] -> wb_prog_delay/5(load_val)
  wb_prog_delay/2(data_out) -> [delay_d0]
  wb_prog_delay/3(dvalid) -> [delay_dv]
  [time80_tl_cd0] -> Slice9/1
  Slice9/1 -> time_gen_cd/3(wr_val_in)
  Constant72/1 -> Concat7/2
  time_gen_cd/3(rd_val_out) -> Concat7/1
  Concat7/1 -> [tg_rd_time_out]
  wb_prog_delay/1(sync_out) -> [delay_sync]
  pipeline300/1(q) -> tl_cd_bs0/1(rst)
  pipeline223/1(q) -> tl_cd_bs0/2(current_time)
  pipeline301/1(q) -> tl_cd_bs0/3(load_value)
  pipeline302/1(q) -> tl_cd_bs0/4(sync_in)
  pipeline303/1(q) -> tl_cd_bs0/5(din)
  pipeline304/1(q) -> tl_cd_bs0/6(en)
  [clr_status] -> pipeline300/1(d)
  [tg_rd_time_out] -> pipeline223/1(d)
  [ld_val0] -> pipeline301/1(d)
  [tg_rd_sync] -> pipeline302/1(d)
  [tg_rd_dvalid] -> pipeline304/1(d)
  Constant71/1 -> tl_cd_bs0/7(misci)
  tl_cd_bs0/7(misco) -> Terminator12/1
  tl_cd_bs0/4(dout) -> [data_tl_bs0]
  tl_cd_bs0/6(time) -> Terminator13/1
  tl_cd_bs0/5(dvalid) -> [dvalid_tl_bs0]
  tl_cd_bs0/1(value) -> [delay_tl_bs0]
  tl_cd_bs0/3(sync_out) -> [delay_tl_sync0]
  tl_cd_bs0/2(enable) -> [en_tl_bs0]
  pipeline224/1(q) -> tl_cd_bs0/8(arm)
  pipeline226/1(q) -> tl_cd_bs0/9(load_immediate)
  pipeline276/1(q) -> tl_cd_bs0/10(load_time)
  [tl_cd0_arm] -> pipeline224/1(d)
  [tl_cd0_load_immediate] -> pipeline226/1(d)
  [tl_cd0_load_time] -> pipeline276/1(d)
  [data_tl_bs0] -> wb_prog_delay/3(din)
  [tg_data_p0] -> pipeline303/1(d)
  pipeline278/1(q) -> tl_cd1/1(rst)
  [ld_val1] -> pipeline279/1(d)
  pipeline277/1(q) -> tl_cd1/2(current_time)
  pipeline279/1(q) -> tl_cd1/3(load_value)
  pipeline283/1(q) -> tl_cd1/4(sync_in)
  pipeline280/1(q) -> tl_cd1/5(din)
  pipeline281/1(q) -> tl_cd1/6(en)
  pipeline284/1(q) -> tl_cd1/9(load_immediate)
  pipeline282/1(q) -> tl_cd1/8(arm)
  pipeline285/1(q) -> tl_cd1/10(load_time)
  [tl_cd1_arm] -> pipeline282/1(d)
  [tl_cd1_load_immediate] -> pipeline284/1(d)
  [tl_cd1_load_time] -> pipeline285/1(d)
  Constant18/1 -> tl_cd1/7(misci)
  tl_cd1/1(value) -> [delay_tl_cd1]
  tl_cd1/2(enable) -> [en_tl_cd1]
  tl_cd1/3(sync_out) -> [sync_tl_cd1]
  tl_cd1/4(dout) -> [dout_tl_cd1]
  tl_cd1/5(dvalid) -> [dvalid_tl_cd1]
  tl_cd1/6(time) -> [time80_tl_cd1]
  tl_cd1/7(misco) -> Terminator22/1
  [delay_tl_cd1] -> Delay111/1
  [en_tl_cd1] -> Delay118/1
  [sync_tl_cd1] -> Delay114/1
  [dvalid_tl_cd1] -> Delay112/1
  pipeline287/1(q) -> tl_cd_bs1/1(rst)
  pipeline286/1(q) -> tl_cd_bs1/2(current_time)
  pipeline288/1(q) -> tl_cd_bs1/3(load_value)
  pipeline289/1(q) -> tl_cd_bs1/4(sync_in)
  pipeline290/1(q) -> tl_cd_bs1/5(din)
  pipeline291/1(q) -> tl_cd_bs1/6(en)
  [clr_status] -> pipeline287/1(d)
  [tg_rd_time_out] -> pipeline286/1(d)
  [ld_val1] -> pipeline288/1(d)
  [tg_rd_sync] -> pipeline289/1(d)
  [tg_rd_dvalid] -> pipeline291/1(d)
  [tg_data_p1] -> pipeline290/1(d)
  Constant73/1 -> tl_cd_bs1/7(misci)
  pipeline225/1(q) -> tl_cd_bs1/8(arm)
  pipeline292/1(q) -> tl_cd_bs1/9(load_immediate)
  pipeline293/1(q) -> tl_cd_bs1/10(load_time)
  [tl_cd1_load_time] -> pipeline293/1(d)
  [tl_cd1_load_immediate] -> pipeline292/1(d)
  [tl_cd1_arm] -> pipeline225/1(d)
  tl_cd_bs1/7(misco) -> Terminator10/1
  tl_cd_bs1/4(dout) -> [data_tl_bs1]
  tl_cd_bs1/6(time) -> Terminator23/1
  tl_cd_bs1/5(dvalid) -> [dvalid_tl_bs1]
  tl_cd_bs1/1(value) -> [delay_tl_bs1]
  tl_cd_bs1/3(sync_out) -> [delay_tl_sync1]
  tl_cd_bs1/2(enable) -> [en_tl_bs1]
  [delay_tl_sync1] -> wb_prog_delay1/1(sync)
  [delay_tl_bs1] -> wb_prog_delay1/2(delay_val)
  [en_tl_bs1] -> wb_prog_delay1/5(load_val)
  [data_tl_bs1] -> wb_prog_delay1/3(din)
  [dvalid_tl_bs1] -> wb_prog_delay1/4(en)
  wb_prog_delay1/2(data_out) -> [delay_d1]
  wb_prog_delay1/1(sync_out) -> Terminator16/1
  wb_prog_delay1/3(dvalid) -> Terminator11/1
  [d80_adc1] -> pipeline280/1(d)
  [hmc_data_p0] -> Concat5/1
  [hmc_data_p1] -> Concat5/2
  bus_expand7/1(msb_out2) -> [tg_data_p0]
  bus_expand7/2(lsb_out1) -> [tg_data_p1]
  Delay120/1 -> Gateway Out43/1, cd_hmc/1(sync_in)
  Delay117/1 -> Gateway Out44/1, cd_hmc/3(en)
  Delay116/1 -> cd_hmc/4(delay_val)
  Delay115/1 -> cd_hmc/5(load_val)
  [dout_tl_cd0] -> Delay119/1
  Delay119/1 -> cd_hmc/2(din)
  [dout_tl_cd1] -> Delay113/1
  Delay114/1 -> cd_hmc/6(sync_in1)
  Delay113/1 -> cd_hmc/7(din1)
  Delay112/1 -> cd_hmc/8(en1)
  Delay111/1 -> cd_hmc/9(delay_val1)
  Delay118/1 -> cd_hmc/10(load_val1)
  [hmc_dvalid] -> Subsystem9/1(In1)
  Subsystem9/1(Out1) -> pipeline274/1(d)
  [dvalid_tl_cd0] -> Subsystem5/1(In1)
  sync_mangle/1(sync_out) -> [hmc_sync_p0]
  sync_mangle/2(dout0) -> [hmc_data_p0]
  sync_mangle/4(dvalid) -> [hmc_dvalid]
  cd_hmc/1(sync_out) -> Delay121/1, sync_mangle/1(sync_in)
  cd_hmc/2(dout0) -> sync_mangle/2(din)
  cd_hmc/4(dvalid) -> sync_mangle/4(en)
  cd_hmc/5(hmc_error) -> [cd_error]
  cd_hmc/3(dout1) -> sync_mangle/3(din1)
  sync_mangle/3(dout1) -> [hmc_data_p1]
  Delay121/1 -> [hmc_sync_raw]
  Subsystem5/1(Out1) -> pipeline275/1(d)
  pipeline274/1(q) -> cd_out_dv_rate/1(out_reg)
  pipeline275/1(q) -> cd_in_dv_rate/1(out_reg)
  Gateway Out43/1 -> To Workspace43/1
  Gateway Out44/1 -> To Workspace44/1
  [cd_bypass] -> Mux15/1
  [cd_bypass] -> Mux13/1
  Mux15/1 -> [pfbin_sync]
  Mux13/1 -> [pfbin_dv]
  [cd_bypass] -> Mux14/1
  Mux14/1 -> [pfbin_d0]
  [cd_bypass] -> Mux16/1
  Mux16/1 -> [pfbin_d1]
  [delay_sync] -> Mux15/2
  [sync80] -> Mux15/3
  [delay_d0] -> Mux14/2
  [d80_adc0] -> Mux14/3
  [delay_d1] -> Mux16/2
  [d80_adc1] -> Mux16/3
  [valid80] -> Mux13/3
  [delay_dv] -> Mux13/2
  pipeline4/1(q) -> sync_status0/1(out_synced_delay)
  pipeline5/1(q) -> sync_status0/2(out_board_in_fault)
  pipeline10/1(q) -> sync_status0/3(out_synced)
  pipeline11/1(q) -> sync_status0/4(out_sync_armed)
  pipeline16/1(q) -> sync_status0/5(out_sync_ct)
  pipeline20/1(q) -> sync_status0/6(out_sync_flag)
  pipeline21/1(q) -> sync_status0/7(out_sync_pack)
  pipeline25/1(q) -> sync_status1/1(out_timeout_resync_cnt)
  pipeline26/1(q) -> sync_status1/2(out_time_diff_resync_cnt)
  pipeline27/1(q) -> sync_status1/3(out_timestep_resync_cnt)
  pipeline32/1(q) -> sync_status1/4(out_cd_resync_cnt)
  pipeline33/1(q) -> sync_status1/5(out_ct_resync_cnt)
  pipeline34/1(q) -> sync_status2/1(out_sync80_cnt)
  pipeline35/1(q) -> sync_status2/2(out_sync_pfb_cnt)
  pipeline36/1(q) -> sync_status2/3(out_sync_fdfs_cnt)
  pipeline37/1(q) -> sync_status2/4(out_sync_quant_cnt)
  pipeline40/1(q) -> unpack_status/1(out_magic_err_cnt)
  pipeline44/1(q) -> unpack_status/2(out_header_err_cnt)
  pipeline61/1(q) -> unpack_status/3(out_pad_err_cnt)
  pipeline80/1(q) -> unpack_status/4(out_pkt_len_err_cnt)
  pipeline82/1(q) -> unpack_status/5(out_time_err_cnt)
  pipeline84/1(q) -> unpack_status1/1(out_pkt_cnt)
  pipeline85/1(q) -> reorder_status/1(out_timestep_err_cnt)
  pipeline86/1(q) -> reorder_status/2(out_receive_err_cnt)
  pipeline87/1(q) -> reorder_status/3(out_discard_cnt)
  pipeline88/1(q) -> reorder_status/4(out_relock_err_cnt)
  pipeline89/1(q) -> reorder_status/5(out_overflow_err_cnt)
  pipeline90/1(q) -> reorder_status/6(out_dv_cnt)
  pipeline91/1(q) -> reorder_status1/1(out_dv_per_sec)
  pipeline92/1(q) -> DDC/7(osc1)
  pipeline93/1(q) -> DDC/6(pol1_data)
  pipeline94/1(q) -> DDC/4(osc0)
  pipeline95/1(q) -> DDC/3(pol0_data)
  pipeline96/1(q) -> DDC/2(dvalid)
  pipeline97/1(q) -> DDC/1(sync)
  pipeline99/1(q) -> Counter3/1
  pipeline105/1(q) -> Counter3/2
  pipeline106/1(q) -> Counter5/1
  pipeline119/1(q) -> Counter5/2
  pipeline126/1(q) -> Counter7/2
  pipeline129/1(q) -> Counter7/1
  pipeline130/1(q) -> [ld_val_fd1]
  pipeline133/1(q) -> [ld_val_fd0]
  we_delay1/1(q) -> [ct_error]
  we_delay2/1(q) -> [ct_pkt_x_offset]
  we_delay7/1(q) -> [ct_pkt_x_index]
  we_delay8/1(q) -> [ct_pktfreq]
  we_delay9/1(q) -> [ct_pktstart]
  we_delay10/1(q) -> [ct_d256]
  we_delay11/1(q) -> [ct_dv]
  we_delay12/1(q) -> [ct_sync]
  we_delay14/1(q) -> hmc_ct/1(in_sync)
  we_delay15/1(q) -> hmc_ct/2(in_dv)
  we_delay16/1(q) -> hmc_ct/3(in_d0)
  we_delay17/1(q) -> hmc_ct/4(in_d1)
  we_delay18/1(q) -> quant_status/1(out_pol0_or_cnt)
  we_delay19/1(q) -> quant_status/2(out_pol1_or_cnt)
  pipeline139/1(q) -> Relational4/2
  pipeline140/1(q) -> time_difference/1(out_time_difference)
  pipeline141/1(q) -> [ld_val1]
  pipeline142/1(q) -> [ld_val0]
  we_delay20/1(q) -> ct_dv_err1/1(out_pktlen_err_cnt)
  we_delay21/1(q) -> ct_dv_err1/2(out_dvblock_err_cnt)
  we_delay22/1(q) -> ct_out_dv_rate1/1(out_reg)
  we_delay23/1(q) -> ct_in_dv_rate1/1(out_reg)
  we_delay24/1(q) -> ct_sync_cnt1/1(out_sync_in)
  we_delay25/1(q) -> ct_sync_cnt1/2(out_rd_addr_arm_cnt)
  we_delay26/1(q) -> ct_sync_cnt1/3(out_rd_sync_cnt)
  we_delay27/1(q) -> ct_sync_cnt1/4(out_sync_out)
  pipeline144/1(q) -> [ddc_sync_in]
  pipeline145/1(q) -> [ddc_dv_in]
  pipeline146/1(q) -> [ddc_pol0_in]
  pipeline149/1(q) -> [ddc_pol1_in]
  Delay2/1 -> pipeline145/1(d)
  Delay9/1 -> pipeline146/1(d)
  pipeline121/1(q) -> local_time_lsw/1(out_timestamp_lsw)
  pipeline123/1(q) -> local_time_msw/1(out_timestamp_msw)
  pipeline124/1(q) -> impulse_gen1/3(impulse_amplitude)
  pipeline125/1(q) -> Slice8/1
  pipeline151/1(q) -> impulse_gen0/3(impulse_amplitude)
  pipeline152/1(q) -> Slice7/1
  we_delay28/1(q) -> quant1/4(coeffs)
  we_delay29/1(q) -> quant0/4(coeffs)
  pipeline155/1(q) -> snap_fft/7(we)
  pipeline154/1(q) -> snap_fft/1(in_real)
  pipeline156/1(q) -> snap_fft/2(in_imag)
  pipeline153/1(q) -> snap_fft/5(in_sync)
  pipeline158/1(q) -> snap_fft/6(in_dv)
  bus_expand3/2(lsb_out1) -> pipeline156/1(d)
  bus_expand3/1(msb_out2) -> pipeline154/1(d)
  generate_pkt_sync2/3(out_data) -> pipeline160/1(d)
  generate_pkt_sync2/2(out_valid) -> pipeline159/1(d)
  generate_pkt_sync2/4(packet_sync) -> pipeline161/1(d)
  [data_pol0] -> generate_pkt_sync2/3(data)
  [fft_sync] -> generate_pkt_sync2/1(sync)
  [fft_dvalid] -> generate_pkt_sync2/2(valid)
  generate_pkt_sync2/1(out_sync) -> Terminator5/1
  pipeline159/1(q) -> pipeline158/1(d), pipeline155/1(d)
  pipeline160/1(q) -> bus_expand3/1(bus_in)
  pipeline161/1(q) -> pipeline153/1(d), snap_fft/8(trig)
  pipeline221/1(q) -> snap_fft/4(in_cap_now)
  pipeline222/1(q) -> snap_fft/3(in_cur_ch)
  [current_channel] -> pipeline222/1(d)
  [capture_now] -> pipeline221/1(d)
  Counter15/1 -> pipeline236/1(d)
  [ddc_sync_in] -> pipeline240/1(d)
  [sys_rst] -> pipeline239/1(d)
  pipeline236/1(q) -> ddc_in_sync_cnt/1(out_reg)
  Counter6/1 -> pipeline163/1(d)
  [sync80] -> Counter6/2
  [sys_rst] -> Counter6/1
  pipeline163/1(q) -> unpack_sync_cnt/1(out_reg)
  pipeline227/1(q) -> snap_ddc_out/5(we)
  pipeline165/1(q) -> snap_ddc_out/4(in_dv)
  pipeline218/1(q) -> snap_ddc_out/3(in_sync)
  [ddc_dvalid] -> pipeline227/1(d), pipeline165/1(d)
  [ddc_sync] -> snap_ddc_out/6(trig), pipeline218/1(d)
  [ddc_pol0] -> pipeline219/1(d)
  pipeline219/1(q) -> snap_ddc_out/1(in_p0)
  pipeline220/1(q) -> snap_ddc_out/2(in_p1)
  [ddc_pol1] -> pipeline220/1(d)
  bus_expand2/1(msb_out8) -> pipeline168/1(d)
  bus_expand2/2(out7) -> pipeline169/1(d)
  bus_expand2/3(out6) -> pipeline190/1(d)
  bus_expand2/4(out5) -> pipeline192/1(d)
  bus_expand2/5(out4) -> pipeline201/1(d)
  bus_expand2/6(out3) -> pipeline203/1(d)
  pipeline168/1(q) -> snap_nb/1(in_real0)
  pipeline169/1(q) -> snap_nb/2(in_imag0)
  pipeline192/1(q) -> snap_nb/4(in_imag1)
  pipeline201/1(q) -> snap_nb/5(in_real2)
  pipeline203/1(q) -> snap_nb/6(in_imag2)
  pipeline232/1(q) -> snap_nb/7(we)
  generate_pkt_sync1/2(out_valid) -> pipeline205/1(d)
  pipeline204/1(q) -> snap_nb1/1(in_real3)
  pipeline217/1(q) -> snap_nb1/2(in_imag3)
  bus_expand2/7(out2) -> pipeline204/1(d)
  bus_expand2/8(lsb_out1) -> pipeline217/1(d)
  pipeline233/1(q) -> snap_nb1/5(we)
  pipeline229/1(q) -> snap_nb1/4(in_dv)
  pipeline230/1(q) -> snap_nb1/3(in_sync)
  generate_pkt_sync1/4(packet_sync) -> pipeline216/1(d)
  pipeline190/1(q) -> snap_nb/3(in_real1)
  [pfb_sync] -> generate_pkt_sync1/1(sync)
  [pfb_dv] -> generate_pkt_sync1/2(valid)
  [pfb_d144_0] -> generate_pkt_sync1/3(data)
  generate_pkt_sync1/3(out_data) -> pipeline206/1(d)
  generate_pkt_sync1/1(out_sync) -> Terminator2/1
  pipeline205/1(q) -> pipeline232/1(d), Gateway Out38/1, pipeline233/1(d), pipeline229/1(d)
  pipeline206/1(q) -> bus_expand2/1(bus_in)
  pipeline216/1(q) -> pipeline230/1(d), Gateway Out39/1, snap_nb1/6(trig), snap_nb/8(trig)
  Gateway Out38/1 -> To Workspace38/1
  Gateway Out39/1 -> To Workspace39/1
  [q_sync] -> Gateway Out36/1, generate_pkt_sync0/1(sync)
  Concat/1 -> generate_pkt_sync0/3(data)
  generate_pkt_sync0/1(out_sync) -> Terminator18/1
  [q_d64_0] -> Concat/1
  [q_d64_1] -> Concat/2
  bus_expand14/1(msb_out2) -> bus_expand12/1(bus_in)
  [q_dv] -> Gateway Out37/1, generate_pkt_sync0/2(valid)
  generate_pkt_sync0/3(out_data) -> pipeline234/1(d)
  generate_pkt_sync0/2(out_valid) -> pipeline235/1(d)
  generate_pkt_sync0/4(packet_sync) -> pipeline237/1(d)
  pipeline234/1(q) -> bus_expand14/1(bus_in)
  pipeline235/1(q) -> Gateway Out31/1, snap_quant0/9(we)
  pipeline237/1(q) -> Gateway Out27/1, snap_quant0/10(trig)
  bus_expand12/1(msb_out8) -> Gateway Out32/1, snap_quant0/1(in_real0)
  bus_expand12/2(out7) -> Gateway Out33/1, snap_quant0/2(in_imag0)
  bus_expand12/3(out6) -> snap_quant0/3(in_real1)
  bus_expand12/4(out5) -> snap_quant0/4(in_imag1)
  bus_expand12/5(out4) -> snap_quant0/5(in_real2)
  bus_expand12/6(out3) -> snap_quant0/6(in_imag2)
  bus_expand12/7(out2) -> Gateway Out34/1, snap_quant0/7(in_real3)
  bus_expand12/8(lsb_out1) -> Gateway Out35/1, snap_quant0/8(in_imag3)
  Counter14/1 -> pipeline238/1(d)
  [q_sync] -> pipeline242/1(d)
  [sys_rst] -> pipeline241/1(d)
  pipeline238/1(q) -> quant_sync_cnt/1(out_reg)
  Gateway Out27/1 -> To Workspace27/1
  Gateway Out31/1 -> To Workspace31/1
  Gateway Out32/1 -> To Workspace32/1
  Gateway Out33/1 -> To Workspace33/1
  Gateway Out34/1 -> To Workspace34/1
  Gateway Out35/1 -> To Workspace35/1
  Gateway Out36/1 -> To Workspace36/1
  Gateway Out37/1 -> To Workspace37/1
  pipeline240/1(q) -> Counter15/2
  pipeline239/1(q) -> Counter15/1
  pipeline241/1(q) -> Counter14/1
  pipeline242/1(q) -> Counter14/2
